simulink model "full_triped"  (R2020a)
settings: Solver=VariableStepAuto  StartTime=0.0  StopTime=10.0  RelTol=1e-3
undefined symbols (not defined in the file): X, Y, Z
libraries: sm_lib x6
settings Model: Creator=jan-b  LastModifiedBy=max

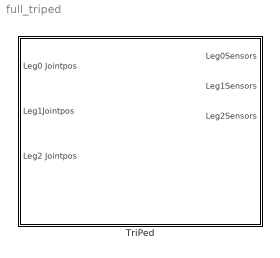
== system full_triped ==
  TriPed : Subsystem
      mask Display: image('./icons/simplified_triped_logo.png')

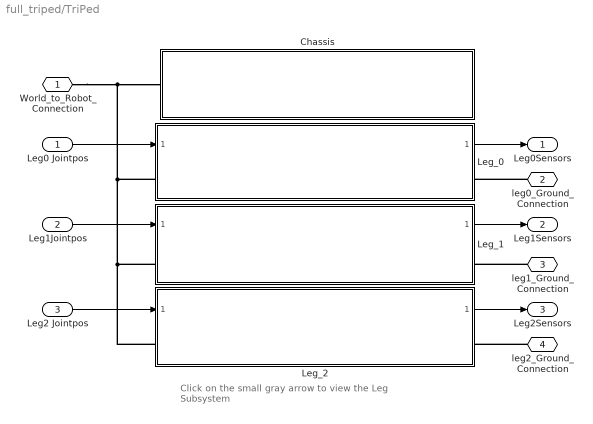
== system full_triped/TriPed ==
  note: Click on the small gray arrow to view the Leg Subsystem
  Leg0 Jointpos : Inport
  Leg1Jointpos : Inport  Port=2
  Leg2 Jointpos : Inport  Port=3
  Chassis : Subsystem
  Leg_0 : Subsystem  ReferencedSubsystem=Full_Leg_Assembly  LegNumber=0
  Leg_1 : Subsystem  ReferencedSubsystem=Full_Leg_Assembly  LegNumber=1
  Leg_2 : Subsystem  ReferencedSubsystem=Full_Leg_Assembly  LegNumber=2
  World_to_Robot_Connection : PMIOPort  Side=Left
  leg0_Ground_Connection : PMIOPort  Port=2  Side=Right
  leg1_Ground_Connection : PMIOPort  Port=3  Side=Right
  leg2_Ground_Connection : PMIOPort  Port=4  Side=Right
  Leg0Sensors : Outport
  Leg1Sensors : Outport  Port=2
  Leg2Sensors : Outport  Port=3
  wires:
  Leg1Jointpos/1 -> Leg_1/1
  Leg2 Jointpos/1 -> Leg_2/1
  Leg_0/1 -> Leg0Sensors/1
  Leg_1/1 -> Leg1Sensors/1
  Leg_2/1 -> Leg2Sensors/1
  Leg0 Jointpos/1 -> Leg_0/1
  connections:
  Leg_2/LConn1 -- Leg_1/LConn1 -- World_to_Robot_Connection/RConn1 -- Chassis/LConn1 -- Leg_0/LConn1
  leg0_Ground_Connection/RConn1 -- Leg_0/RConn1
  leg1_Ground_Connection/RConn1 -- Leg_1/RConn1
  leg2_Ground_Connection/RConn1 -- Leg_2/RConn1

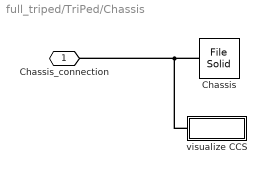
== system full_triped/TriPed/Chassis ==
  Chassis : sm_lib/Body Elements/File Solid  ExtGeomFileName=./triped-files/TriPed_Chassis_überarbeitet.stl  UnitType=Custom  ExtGeomFileUnits=mm  StepReaderType=HEX  ExportConvexHullGeometry=off  InertiaType=Custom  Mass=9.273  MassUnits=kg  CenterOfMass=[0 0 10.44]  CenterOfMassUnits=mm  MomentsOfInertia=[0.121 0.121 0.199]  MomentsOfInertiaUnits=kg*m^2  ProductsOfInertia=[0 0 0]  ProductsOfInertiaUnits=kg*m^2  BasedOnType=DensityFromFile  Density=1000  DensityUnits=kg/m^3  DoExposeReferenceFrame=on  SerializedFrames=<Frames/>  GraphicType=FromGeometry  MarkerShape=Sphere  MarkerSize=10  GraphicVisPropType=SimpleVisualProperties  GraphicDiffuseColor=[1.0 0.63529414 0.0]  GraphicSpecularColor=[0.5 0.5 0.5 1.0]  GraphicAmbientColor=[0.15 0.15 0.15 1.0]  GraphicEmissiveColor=[0.0 0.0 0.0 1.0]  GraphicShininess=75  GraphicOpacity=1
  visualize CCS : Subsystem
  " Chassis_connection" : PMIOPort  Side=Left
  connections:
  Chassis/RConn1 -- visualize CCS/LConn1 -- " Chassis_connection"/RConn1

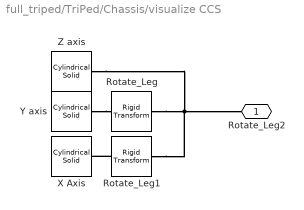
== system full_triped/TriPed/Chassis/visualize CCS ==
  Rotate_Leg : sm_lib/Frames and Transforms/Rigid Transform  TranslationMethod=None  TranslationStandardOffset=0  TranslationLengthUnit=m  TranslationStandardAxis=+Z  TranslationCartesianOffset=[0 0 0]  TranslationROffset=0  TranslationROffsetUnits=m  TranslationZOffset=0  TranslationZOffsetUnits=m  TranslationThetaOffset=0  TranslationThetaOffsetUnits=deg  RotationMethod=StandardAxis  RotationAngleUnits=deg  RotationStandardAxis=+X  RotationAngle=90  RotationArbitraryAxis=[0 0 1]  FollAlignAxisA=+X  BaseAlignAxisA=+Y  FollAlignAxisB=+Y  BaseAlignAxisB=+Z  RotationSequenceAxes=FollowerAxes  RotationSequence=XYX  RotationSequenceAngles=[0 0 0]  RotationSequenceAnglesUnits=deg  RotationMatrix=[1 0 0; 0 1 0; 0 0 1]
  Rotate_Leg1 : sm_lib/Frames and Transforms/Rigid Transform  TranslationMethod=None  TranslationStandardOffset=0  TranslationLengthUnit=m  TranslationStandardAxis=+Z  TranslationCartesianOffset=[0 0 0]  TranslationROffset=0  TranslationROffsetUnits=m  TranslationZOffset=0  TranslationZOffsetUnits=m  TranslationThetaOffset=0  TranslationThetaOffsetUnits=deg  RotationMethod=StandardAxis  RotationAngleUnits=deg  RotationStandardAxis=+Y  RotationAngle=90  RotationArbitraryAxis=[0 0 1]  FollAlignAxisA=+X  BaseAlignAxisA=+Y  FollAlignAxisB=+Y  BaseAlignAxisB=+Z  RotationSequenceAxes=FollowerAxes  RotationSequence=XYX  RotationSequenceAngles=[0 0 0]  RotationSequenceAnglesUnits=deg  RotationMatrix=[1 0 0; 0 1 0; 0 0 1]
  X Axis : sm_lib/Body Elements/Cylindrical Solid  GraphicAmbientColor=[0.15 0.15 0.15 1.0]  BasedOnType=Density  CenterOfMass=[0 0 0]  CenterOfMassUnits=m  GraphicDiffuseColor=[1.0 0.0 0.0]  CylinderLength=0.8  CylinderLengthUnits=m  CylinderRadius=0.01  CylinderRadiusUnits=m  Density=1  DensityUnits=kg/m^3  DoExposeReferenceFrame=on  GraphicEmissiveColor=[0.0 0.0 0.0 1.0]  ExportEntireGeometry=off  SerializedFrames=<Frames/>  MarkerShape=Sphere  MarkerSize=10  GraphicType=FromGeometry  InertiaType=CalculateFromGeometry  Mass=1  MassUnits=kg  MomentsOfInertia=[1 1 1]  MomentsOfInertiaUnits=kg*m^2  GraphicOpacity=1.0  ProductsOfInertia=[0 0 0]  ProductsOfInertiaUnits=kg*m^2  GraphicShininess=75  GraphicSpecularColor=[0.5 0.5 0.5 1.0]  GraphicVisPropType=SimpleVisualProperties
  Y axis : sm_lib/Body Elements/Cylindrical Solid  GraphicAmbientColor=[0.15 0.15 0.15 1.0]  BasedOnType=Density  CenterOfMass=[0 0 0]  CenterOfMassUnits=m  GraphicDiffuseColor=[0.0 1.0 0.0]  CylinderLength=0.8  CylinderLengthUnits=m  CylinderRadius=0.01  CylinderRadiusUnits=m  Density=1  DensityUnits=kg/m^3  DoExposeReferenceFrame=on  GraphicEmissiveColor=[0.0 0.0 0.0 1.0]  ExportEntireGeometry=off  SerializedFrames=<Frames/>  MarkerShape=Sphere  MarkerSize=10  GraphicType=FromGeometry  InertiaType=CalculateFromGeometry  Mass=1  MassUnits=kg  MomentsOfInertia=[1 1 1]  MomentsOfInertiaUnits=kg*m^2  GraphicOpacity=1.0  ProductsOfInertia=[0 0 0]  ProductsOfInertiaUnits=kg*m^2  GraphicShininess=75  GraphicSpecularColor=[0.5 0.5 0.5 1.0]  GraphicVisPropType=SimpleVisualProperties
  Z axis : sm_lib/Body Elements/Cylindrical Solid  GraphicAmbientColor=[0.15 0.15 0.15 1.0]  BasedOnType=Density  CenterOfMass=[0 0 0]  CenterOfMassUnits=m  GraphicDiffuseColor=[0.0 0.0 1.0]  CylinderLength=0.8  CylinderLengthUnits=m  CylinderRadius=0.01  CylinderRadiusUnits=m  Density=1  DensityUnits=kg/m^3  DoExposeReferenceFrame=on  GraphicEmissiveColor=[0.0 0.0 0.0 1.0]  ExportEntireGeometry=off  SerializedFrames=<Frames/>  MarkerShape=Sphere  MarkerSize=10  GraphicType=FromGeometry  InertiaType=CalculateFromGeometry  Mass=1  MassUnits=kg  MomentsOfInertia=[1 1 1]  MomentsOfInertiaUnits=kg*m^2  GraphicOpacity=1.0  ProductsOfInertia=[0 0 0]  ProductsOfInertiaUnits=kg*m^2  GraphicShininess=75  GraphicSpecularColor=[0.5 0.5 0.5 1.0]  GraphicVisPropType=SimpleVisualProperties
  Rotate_Leg2 : PMIOPort  Side=Left
  connections:
  Z axis/RConn1 -- Rotate_Leg1/RConn1 -- Rotate_Leg2/RConn1 -- Rotate_Leg/RConn1
  Y axis/RConn1 -- Rotate_Leg/LConn1
  Rotate_Leg1/LConn1 -- X Axis/RConn1
